simulink model "ofdm_txrx_supermimo"  (Simulink 7.1)
settings: Solver=VariableStepDiscrete  SolverMode=SingleTasking  StartTime=0.0  StopTime=simOnly_simLength  RelTol=1e-3
PostLoadFcn:
  | ofdm_rx_supermimo_init
InitFcn:
  | ofdm_rx_supermimo_init
StartFcn:
  | ofdm_rx_supermimo_init
values from neighbouring files (symbols this model uses):
  Action0_Config = 0* 31*2^18 + 0*2^24 + PHY_AUTORESPONSE_REQ_GOODHDR + PHY_AUTORESPONSE_REQ_GOODPKT + PHY_AUTORESPONSE_REQ_FLAGA    [ofdm_tx_supermimo_init.m]
  Action1_Config = 0* 62*2^18 + PHY_AUTORESPONSE_REQ_GOODHDR + PHY_AUTORESPONSE_REQ_GOODPKT    [ofdm_tx_supermimo_init.m]
  Action2_Config = 0    [ofdm_tx_supermimo_init.m]
  Action3_Config = 0    [ofdm_tx_supermimo_init.m]
  Action4_Config = 0    [ofdm_tx_supermimo_init.m]
  Action5_Config = 0    [ofdm_tx_supermimo_init.m]
  ActionID_AFTransmit = 31    [ofdm_tx_supermimo_init.m]
  ActionID_Disabled = 0    [ofdm_tx_supermimo_init.m]
  ActionID_SetFlagA = 62    [ofdm_tx_supermimo_init.m]
  ActionID_SetFlagB = 61    [ofdm_tx_supermimo_init.m]
  BER_RAM_init_size = 2048    [ofdm_tx_supermimo_init.m]
  CPLength = 16    [ofdm_tx_supermimo_init.m]
  longCorr_coef_i = [-4*real(LTS) 0]    [ofdm_rx_supermimo_init.m]
  longCorr_coef_q = [4*imag(LTS) 0]    [ofdm_rx_supermimo_init.m]
  Match0_Config = 0    [ofdm_tx_supermimo_init.m]
  Match1_Config = 0    [ofdm_tx_supermimo_init.m]
  Match2_Config = 0    [ofdm_tx_supermimo_init.m]
  Match3_Config = 0    [ofdm_tx_supermimo_init.m]
  Match4_Config = 0    [ofdm_tx_supermimo_init.m]
  Match5_Config = 0    [ofdm_tx_supermimo_init.m]
  Match6_Config = 0    [ofdm_tx_supermimo_init.m]
  Match7_Config = 0    [ofdm_tx_supermimo_init.m]
  max_CP_length = 16    [ofdm_tx_supermimo_init.m]
  max_num_baseRateSymbols = 31    [ofdm_tx_supermimo_init.m]
  max_num_subcarriers = 64    [ofdm_tx_supermimo_init.m]
  max_num_trainingSymbols = 15    [ofdm_tx_supermimo_init.m]
  max_numBytes = 16384    [ofdm_tx_supermimo_init.m]
  max_OFDM_symbols = 511    [ofdm_tx_supermimo_init.m]
  modConstellation_qam16 = .75*[1 3 -1 -3]./3    [ofdm_tx_supermimo_init.m]
  modConstellation_qam64 = 0.875*[3 1 5 7 -3 -1 -5 -7]./7    [ofdm_tx_supermimo_init.m]
  modConstellation_qpsk = [1 -1]./sqrt(2)    [ofdm_tx_supermimo_init.m]
  numBaseRateSymbols = ceil(numHeaderBytes / numBytes_BaseRateOFDMSymbol)    [ofdm_tx_supermimo_init.m]
  numSubcarriers = 64    [ofdm_tx_supermimo_init.m]
  numTrainingSymbols = 2    [ofdm_tx_supermimo_init.m]
  pilot_indicies = 7 + ( (64-21) * 2^8) + ( (64-7) * 2^16) + (21 * 2^24)    (= 356068103)    [ofdm_tx_supermimo_init.m]
  pilotValues = hex2dec('A57D') + (2^16 * hex2dec('5A82'))    [ofdm_tx_supermimo_init.m]
  pkt_crossCorr_thresh = 0.7    [ofdm_rx_supermimo_init.m]
  pkt_energy_thresh = 0    [ofdm_rx_supermimo_init.m]
  pktByteNums = numHeaderBytes +  				byteIndex_numPayloadBytes(1)*2^8 +  				byteIndex_numPayloadBytes(2)*2^16 +  				byteIndex_simpleDynModMasks * 2^24    [ofdm_rx_supermimo_init.m]
  PktDet_Delay = 58+32 +      Symbol_Timing_Offset*2^7 +      0*2^16 +      (117* 2^24)    (= 1962935642)    [ofdm_rx_supermimo_init.m]
  pktTiming_count_payloadStart = pktTiming_count_lsStart + pktTiming_ls_num*pktTiming_ls_length    [ofdm_rx_supermimo_init.m]
  preamble = preamble_scale*[shortsyms_10 longsyms_2]    [ofdm_tx_supermimo_init.m]
  preamble_ROM = preamble_I_ROM + preamble_Q_ROM*2^16    [ofdm_tx_supermimo_init.m]
  RAM_init_size = max_numBytes    (= 16384)    [ofdm_tx_supermimo_init.m]
  reg_AF_Tx_Blanking = (320+80) + ( (320+80+79)*2^16)    (= 31392144)    [ofdm_rx_supermimo_init.m]
  reg_AF_Tx_Scaling = 2944    [ofdm_rx_supermimo_init.m]
  reg_Interrupt_PktBuf_Control = reg_InterruptControl +  	1 * 2^16 +  	0 * 2^24    (= 65656)    [ofdm_rx_supermimo_init.m]
  reg_PilotCalcParams = (4 * 2^16) + (minPilotChanMag)    (= 262184.96)    [ofdm_rx_supermimo_init.m]
  regRx_chanEst_minMag = ((0.5)*2^15) + ((0.5 * 2^15)*2^16)    (= 1073758208)    [ofdm_rx_supermimo_init.m]
  regRx_coarseCalcCorrection = round((1.3e-3 - 1.2982e-3)*2^32)    [ofdm_rx_supermimo_init.m]
  regRx_pilotCalcCorrection = round(1.00115 * 2^31)    [ofdm_rx_supermimo_init.m]
  regRx_preCFOoptions = 0 * 2^0 +  	1 * 2^1 +  	0    (= 2)    [ofdm_rx_supermimo_init.m]
  rx_controlBits = 1 * 2  		 + 1 * 4  		 + 0 * 8  		 + 1 * 16 * rx_SISO_Mode  		 + 1 * 32  		 + 1 * 64  		 + 1 * 128  		 + 1 * 256  		 + 1 * 512  		 + 1 * 1024  		 + 1 * 4096  		 + 0 * 16384  		 + 0 * 32768  		 + 1 * 65536  		 + 0 * 131072  		 + 0 * 262144  		 + 1 * 524288  		 + 1 * 1048576 * tx_Alamouti_Mode  		 + 0 * 2097152           + 1 * 33554432  		 + 0 * 4194304  		 + 0 * 8388608  		 + 0 * 16777216  		 + 1 *  ...(+49 chars)    [ofdm_rx_supermimo_init.m]
  Rx_PktDet_LongCorr_Params = LongCorrCounterLoad + 0*2^8 + 0*2^16 + 255*2^24    (= 4278190325)    [ofdm_rx_supermimo_init.m]
  Rx_PktDet_LongCorrThresholds = 8000    [ofdm_rx_supermimo_init.m]
  rx_postEq_scaling = round(rxScaling*2^11) * (1 + 2^16)    [ofdm_rx_supermimo_init.m]
  RxDataScrambling_Seq = [40 198 78 63 82 173 102 245 48 111 172 115 147 230 216 93 72 65 62 2 205 242 122 90 128 83 105 97 73 10 5 252]    [ofdm_tx_supermimo_init.m]
  RxReg_FixedPktLen = 0    [ofdm_rx_supermimo_init.m]
  shortSymbol_time = shortSymbol_time(1:16).'    [ofdm_tx_supermimo_init.m]
  simOnly_numSamples = length(preamble)+( (numSubcarriers+CPLength)*(numTrainingSymbols + numBaseRateSymbols + numFullRateSymbols/2) )    [ofdm_tx_supermimo_init.m]
  train = [train train]    [ofdm_tx_supermimo_init.m]
  tx_controlBits = (antB_preambleShift * 2^4) + (2^1 * tx_Alamouti_Mode) +  	(2^0 * tx_SISO_Mode) + 4*0 + 8*1 + 0*256 + 512*0 + 1024*0 +  	0*hex2dec('1000') + 0*hex2dec('2000') + 0*hex2dec('4000') +  	1*hex2dec('8000') + 1*hex2dec('10000') + hex2dec('20000') +  	16*hex2dec('100000')    [ofdm_tx_supermimo_init.m]
  tx_delays = (50*2^0) + (0*2^8) + (30*2^12)    (= 122930)    [ofdm_tx_supermimo_init.m]
  tx_scaling = (3072) + (12288 * 2^16)    (= 805309440)    [ofdm_tx_supermimo_init.m]
  TxDataScrambling_Seq = [40 198 78 63 82 173 102 245 48 111 172 115 147 230 216 93 72 65 62 2 205 242 122 90 128 83 105 97 73 10 5 252]    [ofdm_tx_supermimo_init.m]
  txfilt_firpm = 0.95*txfilt_firpm./(max(txfilt_firpm))    [ofdm_tx_supermimo_init.m]
  txfilt_intfilt = [0 intfilt(4,4,.8)]    [ofdm_tx_supermimo_init.m]
  txReg_symbolCounts = (2^8 *numBaseRateSymbols ) + numTrainingSymbols    [ofdm_tx_supermimo_init.m]
  TxRx_FFTScaling = (3 * 2^0) +  	(2 * 2^2) +  	(1 * 2^4) +  	(1 * 2^6) +  	(1 * 2^8) +  	(0 * 2^10)    (= 347)    [ofdm_tx_supermimo_init.m]
undefined symbols (not defined in the file or in its folder): AntA, AntB, BaseRate, Down, gcbh, numBuffs, numBytes, Up, xlBlockLoadCallback, xlGetSimulinkPeriod, xlipgetpartsetting
libraries: xbsIndex_r4 x6035, simulink x4, dspsigops x4, commchan3 x2, dspsrcs4 x2, commrflib2 x2
(part 13 of 61)

== system ofdm_txrx_supermimo/OFDM Rx MIMO/FFT & Chan Est/Addr_Generator ==
  note: The FFT core begins requesting input samples immediately
      | after its Start input is asserted. If Start is asserted again before
      | the first transform is complete, a second transform begins
      | immediately after the first. This is how we sequence the pairs
      | of transforms for the incoming OFDM symbols multiplexed between
      | antennas. The FFT is started as soon as the incoming sample stream
      | passes sample number (numSubcarriers/2)+symbolTimingOffset.
      | This is the lowest latency scheme, loading the FFT core with the
      | input OFDM symbol exactly 1 clock cycle after it's available.
  note: The memory read address is the FFT input index plus an offset in [0,max_cyclic_prefix]. This is how we strip
      | the cyclic prefix from the incoming sample stream- the FFT only gets numSubcarrier samples.
  Payload : Inport  OutDataType=sfix(16)  OutScaling=2^0
  FFT Xn_index : Inport  Port=2  OutDataType=sfix(16)  OutScaling=2^0
  AddSub : xbsIndex_r4/AddSub  mode=Addition  use_carryin=off  use_carryout=off  en=off  latency=0  precision=User Defined  arith_type=Unsigned  n_bits=ceil(log2(max_num_subcarriers))+1  bin_pt=0  quantization=Truncate  overflow=Wrap  dbl_ovrd=off  use_behavioral_HDL=on  pipelined=off  use_rpm=on  hw_selection=Fabric  block_type=addsub
      settings: xl_area=[4 0 0 6 0 0 0]  block_version=VER_STRING_GOES_HERE
  Assert : xbsIndex_r4/Assert  Orientation=left  assert_type=on  type_source=Explicitly  arith_type=Boolean  n_bits=16  bin_pt=14  assert_rate=off  rate_source=Explicitly  period=1  output_port=on  block_type=assert
      settings: infoedit=Asserts a user-defined sample rate and/or type on a signal.<P><P>Hardware notes: In hardware this block costs nothing.  block_version=VER_STRING_GOES_HERE
  Convert : xbsIndex_r4/Convert  arith_type=Unsigned  n_bits=ceil(log2(max_num_subcarriers))+1  bin_pt=0  quantization=Truncate  overflow=Wrap  latency=0  dbl_ovrd=off  pipeline=off  block_type=convert
      settings: block_version=VER_STRING_GOES_HERE
  Delay : xbsIndex_r4/Delay  en=off  latency=64  dbl_ovrd=off  reg_retiming=off  block_type=delay
      settings: infoedit=Hardware notes: A delay line is a chain, each link of which is an SRL16 followed by a flip-flop. If register retiming is enabled, the delay line is a chain of flip-flops.  block_version=VER_STRING_GOES_HERE
  Down Sample : xbsIndex_r4/Down Sample  Orientation=left  sample_ratio=2  sample_phase="Last Value of Frame  (most efficient)"  en=off  latency=1  dbl_ovrd=off  block_type=dsamp
      settings: block_version=VER_STRING_GOES_HERE
  Initial Read Addr Timing Calc : Subsystem
  Inverter : xbsIndex_r4/Inverter  Orientation=left  en=off  latency=0  dbl_ovrd=off  block_type=inv
      settings: block_version=VER_STRING_GOES_HERE
  Inverter1 : xbsIndex_r4/Inverter  en=off  latency=0  dbl_ovrd=off  block_type=inv
      settings: block_version=VER_STRING_GOES_HERE
  Logical1 : xbsIndex_r4/Logical  logical_function=OR  inputs=3  en=off  latency=0  precision=Full  arith_type=Unsigned  n_bits=16  bin_pt=0  align_bp=on  dbl_ovrd=off  block_type=logical
      settings: block_version=VER_STRING_GOES_HERE
  Logical2 : xbsIndex_r4/Logical  logical_function=OR  inputs=2  en=off  latency=0  precision=Full  arith_type=Unsigned  n_bits=16  bin_pt=0  align_bp=on  dbl_ovrd=off  block_type=logical
      settings: block_version=VER_STRING_GOES_HERE
  Logical3 : xbsIndex_r4/Logical  logical_function=OR  inputs=2  en=off  latency=0  precision=Full  arith_type=Unsigned  n_bits=16  bin_pt=0  align_bp=on  dbl_ovrd=off  block_type=logical
      settings: block_version=VER_STRING_GOES_HERE
  Logical4 : xbsIndex_r4/Logical  logical_function=AND  inputs=3  en=off  latency=0  precision=Full  arith_type=Unsigned  n_bits=16  bin_pt=0  align_bp=on  dbl_ovrd=off  block_type=logical
      settings: block_version=VER_STRING_GOES_HERE
  Logical5 : xbsIndex_r4/Logical  logical_function=OR  inputs=2  en=off  latency=0  precision=Full  arith_type=Unsigned  n_bits=16  bin_pt=0  align_bp=on  dbl_ovrd=off  block_type=logical
      settings: block_version=VER_STRING_GOES_HERE
  MSB : xbsIndex_r4/Slice  nbits=1  boolean_output=on  mode=Upper Bit Location + Width  bit1=0  base1=MSB of Input  bit0=0  base0=LSB of Input  dbl_ovrd=off  block_type=slice
      settings: infoedit="Extracts a given range of bits from each input sample and presents it at the output.  The output type is ordinarily unsigned with binary point at zero, but can be Boolean when the slice is one bit wide.<P><P>Hardware notes: In hardware this block costs nothing."  block_version=VER_STRING_GOES_HERE
  Oscillator : Subsystem
  Posedge : Subsystem
  Register : xbsIndex_r4/Register  init=0  rst=on  en=on  dbl_ovrd=off  block_type=register
      settings: block_version=VER_STRING_GOES_HERE
  Relational : xbsIndex_r4/Relational  Orientation=left  mode=a=b  en=off  latency=0  dbl_ovrd=off  block_type=relational
      settings: block_version=VER_STRING_GOES_HERE
  Relational1 : xbsIndex_r4/Relational  mode=a=b  en=off  latency=0  dbl_ovrd=off  block_type=relational
      settings: block_version=VER_STRING_GOES_HERE
  Samp Counter : xbsIndex_r4/Counter  cnt_type=Free Running  cnt_to=1  operation=Up  start_count=0  cnt_by_val=1  arith_type=Unsigned  n_bits=ceil(log2(max_num_subcarriers + max_CP_length))  bin_pt=0  load_pin=off  rst=on  en=on  explicit_period=off  period=1  dbl_ovrd=off  use_behavioral_HDL=off  use_rpm=on  implementation=Fabric  block_type=counter
      settings: xl_area=[5 7 0 9 0 0 0]  block_version=VER_STRING_GOES_HERE
  Up Sample1 : xbsIndex_r4/Up Sample  sample_ratio=4  copy_samples=on  dbl_ovrd=off  block_type=usamp
      settings: infoedit="Up samples input data.  Inserted values  can be zeros or copies of the most recent input sample.<P><P>Hardware notes: No hardware is needed if inserted values are copies of the input sample; otherwise, a mux and single bit flip-flop are used."  block_version=VER_STRING_GOES_HERE
  Up Sample2 : xbsIndex_r4/Up Sample  sample_ratio=4  copy_samples=on  dbl_ovrd=off  block_type=usamp
      settings: infoedit="Up samples input data.  Inserted values  can be zeros or copies of the most recent input sample.<P><P>Hardware notes: No hardware is needed if inserted values are copies of the input sample; otherwise, a mux and single bit flip-flop are used."  block_version=VER_STRING_GOES_HERE
  Up Sample6 : xbsIndex_r4/Up Sample  sample_ratio=2  copy_samples=on  dbl_ovrd=off  block_type=usamp
      settings: infoedit="Up samples input data.  Inserted values  can be zeros or copies of the most recent input sample.<P><P>Hardware notes: No hardware is needed if inserted values are copies of the input sample; otherwise, a mux and single bit flip-flop are used."  block_version=VER_STRING_GOES_HERE
  negedge : Subsystem
  negedge1 : Subsystem
  Write Addr : Outport  OutDataType=sfix(16)  OutScaling=2^0
  Read Addr : Outport  Port=2  OutDataType=sfix(16)  OutScaling=2^0
  Ant Sel : Outport  Port=3  OutDataType=sfix(16)  OutScaling=2^0
  Wr Slot : Outport  Port=4  OutDataType=sfix(16)  OutScaling=2^0
  FFT Start : Outport  Port=5  OutDataType=sfix(16)  OutScaling=2^0
  wires:
  negedge1/1(Q) -> Logical1/1
  Up Sample2/1 -> Logical4/2
  Logical4/1 -> FFT Start/1
  [localReset] -> Register/2
  Assert/1 -> Inverter/1
  Inverter/1 -> Register/1
  Register/1 -> Assert/1, Ant Sel/1
  negedge/1(Q) -> Register/3
  MSB/1 -> negedge/1(D)
  Logical2/1 -> Logical4/3
  Up Sample1/1 -> Relational1/1
  FFT Xn_index/1 -> Convert/1, MSB/1
  Down Sample/1 -> Relational/1
  Relational1/1 -> Logical2/1, Delay/1
  AddSub/1 -> Read Addr/1
  [rx_global_reset_DV2] -> Logical1/3
  [localReset] -> Logical3/2, Oscillator/2(rst)
  Logical3/1 -> Samp Counter/1
  Payload/1 -> negedge1/1(D), Samp Counter/2, Up Sample2/1, Posedge/1(D)
  Logical1/1 -> [localReset]
  Posedge/1(Q) -> Logical1/2
  Relational/1 -> Logical3/1, Oscillator/1(en)
  Samp Counter/1 -> Up Sample1/1, Write Addr/1, Relational/2
  [regRX_sampsPerSymMinusOne] -> Down Sample/1
  [regRx_symbolTimingOffset] -> Up Sample6/1
  Convert/1 -> AddSub/1
  Up Sample6/1 -> AddSub/2
  Initial Read Addr Timing Calc/1(D) -> Relational1/2
  Delay/1 -> Logical2/2
  Oscillator/1(Q) -> Wr Slot/1, Logical5/2
  Logical5/1 -> Logical4/1
  [regRx_AlamoutiMode] -> Inverter1/1
  Inverter1/1 -> Logical5/1

== system ofdm_txrx_supermimo/OFDM Rx MIMO/FFT & Chan Est/Channel Estimation & Pilot Processing ==
  note: Naming conventions:
      | Antennas are labaled A and B
      | H_A-A is channel between TxA and RxA
      | H_A-B is channel between TxA and RxB
      | H_B-A is channel between TxB and RxA
      | H_B-B is channel between TxB and RxB
  I : Inport  OutDataType=sfix(16)  OutScaling=2^0
  Q : Inport  Port=2  OutDataType=sfix(16)  OutScaling=2^0
  Xk Index : Inport  Port=3  OutDataType=sfix(16)  OutScaling=2^0
  Vin : Inport  Port=4  OutDataType=sfix(16)  OutScaling=2^0
  Sym Index : Inport  Port=5  OutDataType=sfix(16)  OutScaling=2^0
  Est Index : Inport  Port=6  OutDataType=sfix(16)  OutScaling=2^0
  Actual Trainig Window : Subsystem
  Bus Creator : Subsystem
  Chan Est Buffer & Mag Calc : Subsystem
  Delay1 : xbsIndex_r4/Delay  en=off  latency=1  dbl_ovrd=off  reg_retiming=on  block_type=delay
      settings: infoedit=Hardware notes: A delay line is a chain, each link of which is an SRL16 followed by a flip-flop. If register retiming is enabled, the delay line is a chain of flip-flops.  xl_area=[0, 0, 0, 0, 0, 0, 0]  block_version=VER_STRING_GOES_HERE
  Delay{2,4..11} (9 alike) : xbsIndex_r4/Delay  en=off  latency=1  dbl_ovrd=off  reg_retiming=off  block_type=delay
      settings: infoedit=Hardware notes: A delay line is a chain, each link of which is an SRL16 followed by a flip-flop. If register retiming is enabled, the delay line is a chain of flip-flops.  xl_area=[0, 0, 0, 0, 0, 0, 0]  block_version=VER_STRING_GOES_HERE
  Delay3 : xbsIndex_r4/Delay  en=off  latency=1  dbl_ovrd=off  reg_retiming=on  block_type=delay
      settings: infoedit=Hardware notes: A delay line is a chain, each link of which is an SRL16 followed by a flip-flop. If register retiming is enabled, the delay line is a chain of flip-flops.  xl_area=[0, 0, 0, 0, 0, 0, 0]  block_version=VER_STRING_GOES_HERE
  Down Sample : xbsIndex_r4/Down Sample  sample_ratio=2  sample_phase="Last Value of Frame  (most efficient)"  en=off  latency=1  dbl_ovrd=off  block_type=dsamp
      settings: block_version=10.1.2
  Downsamplng : Subsystem
  H_A-A Estimate Buffer : Subsystem
  H_A-B Estimate Buffer : Subsystem
  H_B-A Estimate Buffer : Subsystem
  H_B-B Estimate Buffer : Subsystem
  Inverter : xbsIndex_r4/Inverter  en=off  latency=1  dbl_ovrd=off  block_type=inv
      settings: block_version=VER_STRING_GOES_HERE
  Logical1 : xbsIndex_r4/Logical  logical_function=OR  inputs=2  en=off  latency=0  precision=Full  arith_type=Unsigned  n_bits=8  bin_pt=2  align_bp=on  dbl_ovrd=off  block_type=logical
      settings: xl_area=[1 0 0 1 0 0 0]  block_version=VER_STRING_GOES_HERE
  Logical2 : xbsIndex_r4/Logical  logical_function=OR  inputs=2  en=off  latency=0  precision=Full  arith_type=Unsigned  n_bits=8  bin_pt=2  align_bp=on  dbl_ovrd=off  block_type=logical
      settings: xl_area=[1 0 0 1 0 0 0]  block_version=VER_STRING_GOES_HERE
  Mult by training : Subsystem
  Mux3 : xbsIndex_r4/Mux  inputs=2  en=off  latency=0  precision=Full  arith_type="Signed  (2's comp)"  n_bits=8  bin_pt=2  quantization=Truncate  overflow=Wrap  dbl_ovrd=off  block_type=mux
      settings: xl_area=[0, 0, 0, 0, 0, 0, 0]  block_version=VER_STRING_GOES_HERE
  Pilot Processing : Subsystem
  Training Symbol : xbsIndex_r4/ROM  depth=length(train)/2  initVector=sign(train)  distributed_mem=Block RAM  rst=off  init_reg=0  en=off  latency=1  arith_type="Signed  (2's comp)"  n_bits=2  bin_pt=1  dbl_ovrd=off  optimize=Area  use_rpm=off  block_type=sprom
      settings: xl_area=[0, 0, 0, 0, 0, 0, 0]  block_version=VER_STRING_GOES_HERE
  Training? : Subsystem
  WE Gen : Subsystem
  done{1..9,23} (10 alike) : xbsIndex_r4/Gateway Out  hdl_port=off  timing_constraint=None  locs_specified=off  LOCs={}  block_type=gatewayout
      settings: infoedit="Gateway out block.  Converts Xilinx fixed point inputs into ouputs of type Simulink integer, double, or fixed point.<P><P>Hardware notes:  In hardware these blocks become top level output ports or are discarded, depending on how they are configured."  xl_area=[0 0 0 0 1 0 0]  block_version=VER_STRING_GOES_HERE
  H-I : Outport  OutDataType=sfix(16)  OutScaling=2^0
  H-Q : Outport  Port=2  OutDataType=sfix(16)  OutScaling=2^0
  Phase I : Outport  Port=3  OutDataType=sfix(16)  OutScaling=2^0
  Phase Q : Outport  Port=4  OutDataType=sfix(16)  OutScaling=2^0
  Est Vout : Outport  Port=5  OutDataType=sfix(16)  OutScaling=2^0
  wires:
  Delay5/1 -> H_A-B Estimate Buffer/4(Est_Reset), H_B-B Estimate Buffer/4(Est_Reset), Logical1/2, Logical2/2
  Delay2/1 -> Mux3/3, H_A-A Estimate Buffer/5(FFT_Addr), Pilot Processing/3(Xk_Index), H_B-A Estimate Buffer/5(FFT_Addr)
  Est Index/1 -> Mux3/2, Delay7/1, Chan Est Buffer & Mag Calc/10(SubCar Index)
  Delay10/1 -> Delay11/1
  done23/1 -> ?
  done9/1 -> ?
  done2/1 -> ?
  done8/1 -> ?
  done7/1 -> ?
  done6/1 -> ?
  done3/1 -> ?
  done5/1 -> ?
  done4/1 -> ?
  done1/1 -> ?
  H_B-B Estimate Buffer/2(EstQ) -> Downsamplng/8(BB-Q)
  H_B-B Estimate Buffer/1(EstI) -> Downsamplng/7(BB-I)
  H_B-A Estimate Buffer/4(EstQ) -> Downsamplng/6(BA-Q)
  H_B-A Estimate Buffer/3(EstI) -> Downsamplng/5(BA-I)
  H_A-B Estimate Buffer/2(EstQ) -> Downsamplng/4(AB-Q)
  H_A-B Estimate Buffer/1(EstI) -> Downsamplng/3(AB-I)
  H_A-A Estimate Buffer/4(EstQ) -> Downsamplng/2(AA-Q)
  H_A-A Estimate Buffer/3(EstI) -> Downsamplng/1(AA-I)
  Delay11/1 -> Down Sample/1
  Inverter/1 -> Delay10/1
  Delay7/1 -> done2/1
  Delay9/1 -> WE Gen/1(Vin)
  Vin/1 -> Delay9/1, Actual Trainig Window/1(H Vin)
  Delay8/1 -> Mult by training/2(AI)
  Delay6/1 -> Mult by training/1(AR)
  Delay3/1 -> Mux3/1, WE Gen/3(Train), Actual Trainig Window/2(Train Sym)
  Delay1/1 -> WE Gen/2(Sym Index), Pilot Processing/4(Sym_Index)
  Mult by training/2(PI) -> H_A-B Estimate Buffer/2(Q), H_A-A Estimate Buffer/2(Q), H_B-B Estimate Buffer/2(Q), H_B-A Estimate Buffer/2(Q)
  Training?/2(Training) -> Delay3/1, Inverter/1
  WE Gen/2(Seq1) -> H_A-B Estimate Buffer/3(Est_WE)
  WE Gen/4(Seq3) -> H_B-B Estimate Buffer/3(Est_WE)
  WE Gen/3(Seq2) -> H_B-A Estimate Buffer/3(Est_WE)
  WE Gen/1(Seq0) -> H_A-A Estimate Buffer/3(Est_WE)
  Mux3/1 -> H_B-B Estimate Buffer/5(Xk_Index), H_A-B Estimate Buffer/5(Xk_Index), H_A-A Estimate Buffer/6(EQ_Addr), H_B-A Estimate Buffer/6(EQ_Addr)
  Mult by training/1(PR) -> H_A-B Estimate Buffer/1(I), H_A-A Estimate Buffer/1(I), H_B-A Estimate Buffer/1(I), H_B-B Estimate Buffer/1(I)
  Xk Index/1 -> Training Symbol/1, Delay4/1
  Sym Index/1 -> Training?/1(SynIndex), Delay1/1
  Q/1 -> Delay8/1, Pilot Processing/2(Q)
  I/1 -> Delay6/1, Pilot Processing/1(I)
  Delay4/1 -> Delay2/1
  Training Symbol/1 -> Mult by training/3(BR)
  Down Sample/1 -> Est Vout/1, Chan Est Buffer & Mag Calc/9(Est Vin), done23/1
  Bus Creator/1(I) -> H-I/1
  Bus Creator/2(Q) -> H-Q/1
  Downsamplng/1(" AA-I") -> Bus Creator/1(AA-I), Chan Est Buffer & Mag Calc/1(AA-I), done9/1
  Downsamplng/2(" AA-Q") -> Bus Creator/2(AA-Q), Chan Est Buffer & Mag Calc/2(AA-Q), done4/1
  Downsamplng/3(" AB-I") -> Bus Creator/3(AB-I), Chan Est Buffer & Mag Calc/3(AB-I), done5/1
  Downsamplng/4(" AB-Q") -> Bus Creator/4(AB-Q), Chan Est Buffer & Mag Calc/4(AB-Q), done3/1
  Downsamplng/5(" BA-I") -> Bus Creator/5(BA-I), Chan Est Buffer & Mag Calc/5(BA-I), done6/1
  Downsamplng/6(" BA-Q") -> Bus Creator/6(BA-Q), Chan Est Buffer & Mag Calc/6(BA-Q), done1/1
  Downsamplng/7(" BB-I") -> Bus Creator/7(BB-I), Chan Est Buffer & Mag Calc/7(BB-I), done7/1
  Downsamplng/8(" BB-Q") -> Bus Creator/8(BB-Q), Chan Est Buffer & Mag Calc/8(BB-Q), done8/1
  Training?/1(FirstSym) -> Delay5/1
  Pilot Processing/1(Phase I) -> Phase I/1
  H_A-A Estimate Buffer/1(EstI_fast) -> Pilot Processing/6(HAA_I)
  H_A-A Estimate Buffer/2(EstQ_fast) -> Pilot Processing/7(HAA_Q)
  H_B-A Estimate Buffer/2(EstQ_fast) -> Pilot Processing/9(HBA_Q)
  H_B-A Estimate Buffer/1(EstI_fast) -> Pilot Processing/8(HBA_I)
  Actual Trainig Window/1(Train) -> Pilot Processing/5(Train)
  Pilot Processing/2(Phase Q) -> Phase Q/1
  Logical2/1 -> H_A-A Estimate Buffer/4(Est_Reset)
  [ChanEstMagCheck_MaskAA] -> Logical2/1
  Logical1/1 -> H_B-A Estimate Buffer/4(Est_Reset)
  [ChanEstMagCheck_MaskBA] -> Logical1/1

== system ofdm_txrx_supermimo/OFDM Rx MIMO/FFT & Chan Est/FFT ==
  xn_I : Inport  OutDataType=sfix(16)  OutScaling=2^0
  xn_Q : Inport  Port=2  OutDataType=sfix(16)  OutScaling=2^0
  Start : Inport  Port=3  OutDataType=sfix(16)  OutScaling=2^0
  Constant3 : xbsIndex_r4/Constant  arith_type=Boolean  const=1  n_bits=1  bin_pt=0  explicit_period=off  period=1  equ=P=C  opselect=C  inp2=PCIN>>17  opr=+  inp1=P  carry=CIN  dbl_ovrd=off  block_type=constant
      settings: block_version=VER_STRING_GOES_HERE
  Constant4 : xbsIndex_r4/Constant  arith_type=Boolean  const=1  n_bits=1  bin_pt=0  explicit_period=off  period=1  equ=P=C  opselect=C  inp2=PCIN>>17  opr=+  inp1=P  carry=CIN  dbl_ovrd=off  block_type=constant
      settings: block_version=VER_STRING_GOES_HERE
  Constant6 : xbsIndex_r4/Constant  arith_type=Boolean  const=1  n_bits=1  bin_pt=0  explicit_period=off  period=1  equ=P=C  opselect=C  inp2=PCIN>>17  opr=+  inp1=P  carry=CIN  dbl_ovrd=off  block_type=constant
      settings: block_version=VER_STRING_GOES_HERE
  Convert : xbsIndex_r4/Convert  arith_type="Signed  (2's comp)"  n_bits=16  bin_pt=15  quantization=Truncate  overflow=Flag as error  latency=0  dbl_ovrd=off  pipeline=off  block_type=convert
      settings: block_version=VER_STRING_GOES_HERE
  Convert1 : xbsIndex_r4/Convert  arith_type="Signed  (2's comp)"  n_bits=16  bin_pt=15  quantization=Truncate  overflow=Flag as error  latency=0  dbl_ovrd=off  pipeline=off  block_type=convert
      settings: block_version=VER_STRING_GOES_HERE
  "Fast Fourier Transform 6.0 " : xbsIndex_r4/Fast Fourier Transform 6.0   transform_length=64  implementation_options=pipelined_streaming_io  run_time_configurable_transform_length=off  phase_factor_width=24  scaling_options=scaled  rounding_modes=convergent_rounding  output_ordering=natural_order  cyclic_prefix_insertion=off  en=off  rst=on  ovflo=on  memory_options_data=block_ram  memory_options_phase_factors=block_ram  number_of_stages_using_block_ram_for_data_and_phase_factors=1  memory_options_reorder=block_ram  memory_options_hybrid=off  optimize_for_speed_using_xtreme_dsp_slices=off  fast_complex_mult=off  fast_butterfly=off  target_clock_frequency=250  simulation_type=external_xfix  simulation_model=fftv6_CModel:fftv6_cmodel  ip_name=Fast Fourier Transform  ip_version=6.0  dsptool_ready=true  input_ordering=natural_order  ce=true  sclr=0  wrapper_available=true  port_translation_map={ 'ce' => 'en', 'sclr' => 'rst' }  ipcore_fpga_part=xlipgetpartsetting(gcb, {'virtex4', 'xc4vsx35', '-10', 'ff668'})  target_data_throughput=50  channels=1  input_width=16  block_type=xfft_v6_0
      settings: block_version=10.1.3
  "FreqSelective \"Fading\"" : Subsystem
  Up Sample1 : xbsIndex_r4/Up Sample  sample_ratio=2  copy_samples=on  dbl_ovrd=off  block_type=usamp
      settings: infoedit="Up samples input data.  Inserted values  can be zeros or copies of the most recent input sample.<P><P>Hardware notes: No hardware is needed if inserted values are copies of the input sample; otherwise, a mux and single bit flip-flop are used."  block_version=VER_STRING_GOES_HERE
  Up Sample2 : xbsIndex_r4/Up Sample  sample_ratio=2  copy_samples=on  dbl_ovrd=off  block_type=usamp
      settings: infoedit="Up samples input data.  Inserted values  can be zeros or copies of the most recent input sample.<P><P>Hardware notes: No hardware is needed if inserted values are copies of the input sample; otherwise, a mux and single bit flip-flop are used."  block_version=VER_STRING_GOES_HERE
  xk_I : Outport  OutDataType=sfix(16)  OutScaling=2^0
  xk_Q : Outport  Port=2  OutDataType=sfix(16)  OutScaling=2^0
  xk_Index : Outport  Port=3  OutDataType=sfix(16)  OutScaling=2^0
  Vout : Outport  Port=4  OutDataType=sfix(16)  OutScaling=2^0
  Done : Outport  Port=5  OutDataType=sfix(16)  OutScaling=2^0
  xn_Index : Outport  Port=6  OutDataType=sfix(16)  OutScaling=2^0
  tag scope: FFT_Overflow=global
  wires:
  [Rx_FFTScaling] -> Up Sample2/1
  Constant6/1 -> "Fast Fourier Transform 6.0 "/7
  Convert1/1 -> "Fast Fourier Transform 6.0 "/2
  Convert/1 -> "Fast Fourier Transform 6.0 "/1
  "Fast Fourier Transform 6.0 "/7 -> Vout/1
  "Fast Fourier Transform 6.0 "/4 -> xk_Index/1, "FreqSelective \"Fading\""/3(Ind)
  Start/1 -> "Fast Fourier Transform 6.0 "/3
  "Fast Fourier Transform 6.0 "/3 -> xn_Index/1
  xn_Q/1 -> Convert1/1
  "Fast Fourier Transform 6.0 "/2 -> "FreqSelective \"Fading\""/2(Q)
  "Fast Fourier Transform 6.0 "/1 -> "FreqSelective \"Fading\""/1(I)
  xn_I/1 -> Convert/1
  [rx_global_reset] -> Up Sample1/1
  Constant4/1 -> "Fast Fourier Transform 6.0 "/5
  Constant3/1 -> "Fast Fourier Transform 6.0 "/4
  Up Sample1/1 -> "Fast Fourier Transform 6.0 "/8
  Up Sample2/1 -> "Fast Fourier Transform 6.0 "/6
  "Fast Fourier Transform 6.0 "/8 -> Done/1
  "Fast Fourier Transform 6.0 "/9 -> ?
  "Fast Fourier Transform 6.0 "/10 -> [FFT_Overflow]
  "FreqSelective \"Fading\""/1(" I") -> xk_I/1
  "FreqSelective \"Fading\""/2(" Q") -> xk_Q/1

== system ofdm_txrx_supermimo/OFDM Rx MIMO/FFT & Chan Est/Input Buffers ==
  AntA I : Inport  OutDataType=sfix(16)  OutScaling=2^0
  AntA Q : Inport  Port=2  OutDataType=sfix(16)  OutScaling=2^0
  AntB I : Inport  Port=3  OutDataType=sfix(16)  OutScaling=2^0
  AntB Q : Inport  Port=4  OutDataType=sfix(16)  OutScaling=2^0
  We : Inport  Port=5  OutDataType=sfix(16)  OutScaling=2^0
  Wr Addr : Inport  Port=6  OutDataType=sfix(16)  OutScaling=2^0
  Rd Addr : Inport  Port=7  OutDataType=sfix(16)  OutScaling=2^0
  Ant Sel : Inport  Port=8  OutDataType=sfix(16)  OutScaling=2^0
  Wr Slot : Inport  Port=9  OutDataType=sfix(16)  OutScaling=2^0
  Antenna A RAM : Subsystem
  Antenna B RAM : Subsystem
  Down Sample : xbsIndex_r4/Down Sample  sample_ratio=2  sample_phase="Last Value of Frame  (most efficient)"  en=off  latency=1  dbl_ovrd=off  block_type=dsamp
      settings: block_version=VER_STRING_GOES_HERE
  Mux : xbsIndex_r4/Mux  inputs=2  en=off  latency=1  precision=Full  arith_type=Unsigned  n_bits=16  bin_pt=14  quantization=Truncate  overflow=Wrap  dbl_ovrd=off  block_type=mux
      settings: block_version=VER_STRING_GOES_HERE
  Mux1 : xbsIndex_r4/Mux  inputs=2  en=off  latency=1  precision=Full  arith_type=Unsigned  n_bits=16  bin_pt=14  quantization=Truncate  overflow=Wrap  dbl_ovrd=off  block_type=mux
      settings: block_version=VER_STRING_GOES_HERE
  Mux2 : xbsIndex_r4/Mux  inputs=2  en=off  latency=0  precision=Full  arith_type=Unsigned  n_bits=16  bin_pt=14  quantization=Truncate  overflow=Wrap  dbl_ovrd=off  block_type=mux
      settings: block_version=VER_STRING_GOES_HERE
  Mux3 : xbsIndex_r4/Mux  inputs=2  en=off  latency=0  precision=Full  arith_type=Unsigned  n_bits=16  bin_pt=14  quantization=Truncate  overflow=Wrap  dbl_ovrd=off  block_type=mux
      settings: block_version=VER_STRING_GOES_HERE
  Register : xbsIndex_r4/Register  init=0  rst=off  en=off  dbl_ovrd=off  block_type=register
      settings: block_version=VER_STRING_GOES_HERE
  Register1 : xbsIndex_r4/Register  init=0  rst=off  en=off  dbl_ovrd=off  block_type=register
      settings: block_version=VER_STRING_GOES_HERE
  We Control : Subsystem
  " I" : Outport  OutDataType=sfix(16)  OutScaling=2^0
  " Q" : Outport  Port=2  OutDataType=sfix(16)  OutScaling=2^0
  wires:
  Register1/1 -> " Q"/1
  Register/1 -> " I"/1
  Mux1/1 -> Register1/1
  Mux/1 -> Register/1
  Ant Sel/1 -> Mux/1, Mux1/1
  Antenna B RAM/1(" I") -> Mux/3
  Antenna B RAM/2(" Q") -> Mux1/3
  Antenna A RAM/2(" Q") -> Mux1/2
  Antenna A RAM/1(" I") -> Mux/2
  Rd Addr/1 -> Antenna B RAM/5(Rd Addr), Antenna A RAM/5(Rd Addr)
  Wr Addr/1 -> Antenna B RAM/4(Wr Addr), Antenna A RAM/4(Wr Addr)
  Mux2/1 -> Antenna B RAM/1(I)
  AntB I/1 -> Mux2/2
  AntA I/1 -> Antenna A RAM/1(I), Mux2/3
  AntA Q/1 -> Antenna A RAM/2(Q), Mux3/3
  We Control/1(A_We) -> Antenna A RAM/3(We)
  We Control/2(B_We) -> Antenna B RAM/3(We)
  AntB Q/1 -> Mux3/2
  [regRx_AlamoutiMode] -> Down Sample/1
  Mux3/1 -> Antenna B RAM/2(Q)
  We/1 -> We Control/1(We)
  Wr Slot/1 -> We Control/2(WrSlot)
  Down Sample/1 -> Mux3/1, Mux2/1

== system ofdm_txrx_supermimo/OFDM Rx MIMO/FFT & Chan Est/Output Buffers ==
  I : Inport  OutDataType=sfix(16)  OutScaling=2^0
  Q : Inport  Port=2  OutDataType=sfix(16)  OutScaling=2^0
  Wr Addr : Inport  Port=3  OutDataType=sfix(16)  OutScaling=2^0
  FFT Vout : Inport  Port=4  OutDataType=sfix(16)  OutScaling=2^0
  SymIndex : Inport  Port=5  OutDataType=sfix(16)  OutScaling=2^0
  Rd Addr : Inport  Port=6  OutDataType=sfix(16)  OutScaling=2^0
  AntA Buffer : Subsystem
  AntB Buffer : Subsystem
  Bus Creator1 : BusCreator  Inputs=2
  Bus Creator2 : BusCreator  Inputs=2
  Inverter : xbsIndex_r4/Inverter  en=off  latency=0  dbl_ovrd=off  block_type=inv
      settings: block_version=VER_STRING_GOES_HERE
  LSB : xbsIndex_r4/Slice  nbits=1  boolean_output=on  mode=Upper Bit Location + Width  bit1=0  base1=LSB of Input  bit0=0  base0=LSB of Input  dbl_ovrd=off  block_type=slice
      settings: infoedit="Extracts a given range of bits from each input sample and presents it at the output.  The output type is ordinarily unsigned with binary point at zero, but can be Boolean when the slice is one bit wide.<P><P>Hardware notes: In hardware this block costs nothing."  block_version=VER_STRING_GOES_HERE
  Logical1 : xbsIndex_r4/Logical  logical_function=AND  inputs=2  en=off  latency=0  precision=Full  arith_type=Unsigned  n_bits=8  bin_pt=2  align_bp=on  dbl_ovrd=off  block_type=logical
      settings: xl_area=[0, 0, 0, 0, 0, 0, 0]  block_version=VER_STRING_GOES_HERE
  Logical2 : xbsIndex_r4/Logical  logical_function=AND  inputs=2  en=off  latency=0  precision=Full  arith_type=Unsigned  n_bits=8  bin_pt=2  align_bp=on  dbl_ovrd=off  block_type=logical
      settings: xl_area=[0, 0, 0, 0, 0, 0, 0]  block_version=VER_STRING_GOES_HERE
  " I" : Outport  OutDataType=sfix(16)  OutScaling=2^0
  " Q" : Outport  Port=2  OutDataType=sfix(16)  OutScaling=2^0
  wires:
  Bus Creator2/1 -> " Q"/1
  Bus Creator1/1 -> " I"/1
  Inverter/1 -> Logical2/1
  Q/1 -> AntA Buffer/2(Q), AntB Buffer/2(Q)
  I/1 -> AntA Buffer/1(I), AntB Buffer/1(I)
  Wr Addr/1 -> AntA Buffer/4(Wr Addr), AntB Buffer/4(Wr Addr)
  FFT Vout/1 -> Logical1/2, Logical2/2
  AntA Buffer/2(" Q") -> Bus Creator2/2
  AntA Buffer/1(" I") -> Bus Creator1/2
  AntB Buffer/2(" Q") -> Bus Creator2/1
  AntB Buffer/1(" I") -> Bus Creator1/1
  LSB/1 -> Inverter/1, Logical1/1
  Logical1/1 -> AntB Buffer/3(We)
  Logical2/1 -> AntA Buffer/3(We)
  SymIndex/1 -> LSB/1
  Rd Addr/1 -> AntA Buffer/5(Rd Addr), AntB Buffer/5(Rd Addr)

== system ofdm_txrx_supermimo/OFDM Rx MIMO/FFT & Chan Est/Symbol Counter ==
  note: SR Latch enables counter after the first symbol. This keeps
      | the symbol index at zero after the first FFT finishes so that
      | the symbol index, like xn and xk_index, is zero-indexed.
  FFT Done : Inport  OutDataType=sfix(16)  OutScaling=2^0
  Payload : Inport  Port=2  OutDataType=sfix(16)  OutScaling=2^0
  Counter : xbsIndex_r4/Counter  cnt_type=Free Running  cnt_to=Inf  operation=Up  start_count=0  cnt_by_val=1  arith_type=Unsigned  n_bits=ceil(log2(max_OFDM_symbols+1))  bin_pt=0  load_pin=off  rst=on  en=on  explicit_period=off  period=1  dbl_ovrd=off  use_behavioral_HDL=off  use_rpm=on  implementation=Fabric  block_type=counter
      settings: xl_area=[6 12 0 12 0 0 0]  block_version=VER_STRING_GOES_HERE
  Inverter : xbsIndex_r4/Inverter  en=off  latency=0  dbl_ovrd=off  block_type=inv
      settings: xl_area=[0 0 0 0 0 0 0]  block_version=VER_STRING_GOES_HERE
  Logical1 : xbsIndex_r4/Logical  logical_function=AND  inputs=2  en=off  latency=0  precision=Full  arith_type=Unsigned  n_bits=8  bin_pt=2  align_bp=on  dbl_ovrd=off  block_type=logical
      settings: xl_area=[0, 0, 0, 0, 0, 0, 0]  block_version=VER_STRING_GOES_HERE
  Logical2 : xbsIndex_r4/Logical  logical_function=OR  inputs=2  en=off  latency=0  precision=Full  arith_type=Unsigned  n_bits=8  bin_pt=2  align_bp=on  dbl_ovrd=off  block_type=logical
      settings: xl_area=[4 0 0 4 0 0 0]  block_version=VER_STRING_GOES_HERE
  S-R Latch : Subsystem
  Up Sample : xbsIndex_r4/Up Sample  sample_ratio=4  copy_samples=on  dbl_ovrd=off  block_type=usamp
      settings: infoedit="Up samples input data.  Inserted values  can be zeros or copies of the most recent input sample.<P><P>Hardware notes: No hardware is needed if inserted values are copies of the input sample; otherwise, a mux and single bit flip-flop are used."  block_version=10.1.2
  Up Sample1 : xbsIndex_r4/Up Sample  sample_ratio=2  copy_samples=on  dbl_ovrd=off  block_type=usamp
      settings: infoedit="Up samples input data.  Inserted values  can be zeros or copies of the most recent input sample.<P><P>Hardware notes: No hardware is needed if inserted values are copies of the input sample; otherwise, a mux and single bit flip-flop are used."  block_version=10.1.2
  Sym Index : Outport  OutDataType=sfix(16)  OutScaling=2^0
  wires:
  Payload/1 -> Up Sample/1
  [rx_global_reset] -> Up Sample1/1
  Inverter/1 -> Logical2/1
  Logical2/1 -> S-R Latch/2(R), Counter/1
  S-R Latch/1(D) -> Logical1/2
  Logical1/1 -> Counter/2
  Counter/1 -> Sym Index/1
  FFT Done/1 -> S-R Latch/1(S), Logical1/1
  Up Sample/1 -> Inverter/1
  Up Sample1/1 -> Logical2/2

== system ofdm_txrx_supermimo/OFDM Rx MIMO/Long Corr HP/C1 ==
  x : Inport  OutDataType=sfix(16)  OutScaling=2^0
  AddSub : xbsIndex_r4/AddSub  Orientation=down  mode=Addition  use_carryin=off  use_carryout=off  en=off  latency=0  precision=Full  arith_type=Unsigned  n_bits=8  bin_pt=2  quantization=Truncate  overflow=Wrap  dbl_ovrd=off  use_behavioral_HDL=on  pipelined=off  use_rpm=off  hw_selection=Fabric  block_type=addsub
      settings: xl_area=[3 0 0 4 0 0 0]  block_version=VER_STRING_GOES_HERE
  AddSub{1..7,9..14} (13 alike) : xbsIndex_r4/AddSub  Orientation=down  mode=Addition  use_carryin=off  use_carryout=off  en=off  latency=1  precision=Full  arith_type=Unsigned  n_bits=8  bin_pt=2  quantization=Truncate  overflow=Wrap  dbl_ovrd=off  use_behavioral_HDL=on  pipelined=off  use_rpm=off  hw_selection=Fabric  block_type=addsub
      AddSub1 settings: xl_area=[3 5 0 4 0 0 0]  block_version=VER_STRING_GOES_HERE
      AddSub10 settings: xl_area=[3 5 0 4 0 0 0]  block_version=VER_STRING_GOES_HERE
      AddSub11 settings: xl_area=[3 5 0 4 0 0 0]  block_version=VER_STRING_GOES_HERE
      AddSub12 settings: xl_area=[3 5 0 4 0 0 0]  block_version=VER_STRING_GOES_HERE
      AddSub13 settings: xl_area=[3 6 0 5 0 0 0]  block_version=VER_STRING_GOES_HERE
      AddSub14 settings: xl_area=[3 6 0 5 0 0 0]  block_version=VER_STRING_GOES_HERE
      AddSub2 settings: xl_area=[3 6 0 5 0 0 0]  block_version=VER_STRING_GOES_HERE
      AddSub3 settings: xl_area=[3 5 0 4 0 0 0]  block_version=VER_STRING_GOES_HERE
      AddSub4 settings: xl_area=[4 7 0 6 0 0 0]  block_version=VER_STRING_GOES_HERE
      AddSub5 settings: xl_area=[3 5 0 4 0 0 0]  block_version=VER_STRING_GOES_HERE
      AddSub6 settings: xl_area=[3 6 0 5 0 0 0]  block_version=VER_STRING_GOES_HERE
      AddSub7 settings: xl_area=[3 5 0 4 0 0 0]  block_version=VER_STRING_GOES_HERE
      AddSub9 settings: xl_area=[4 7 0 6 0 0 0]  block_version=VER_STRING_GOES_HERE
  AddSub8 : xbsIndex_r4/AddSub  mode=Addition  use_carryin=off  use_carryout=off  en=off  latency=1  precision=Full  arith_type=Unsigned  n_bits=8  bin_pt=2  quantization=Truncate  overflow=Wrap  dbl_ovrd=off  use_behavioral_HDL=on  pipelined=off  use_rpm=off  hw_selection=Fabric  block_type=addsub
      settings: xl_area=[4 8 0 7 0 0 0]  block_version=VER_STRING_GOES_HERE
  C1 : Subsystem  mask{coef2=h(1:N)}
      mask prompts: coef2 "Coefficients"
  C10 : Subsystem  mask{coef2=h(9*N+1:10*N)}
      mask prompts: coef2 "Coefficients"
  C11 : Subsystem  mask{coef2=h(10*N+1:11*N)}
      mask prompts: coef2 "Coefficients"
  C12 : Subsystem  mask{coef2=h(11*N+1:12*N)}
      mask prompts: coef2 "Coefficients"
  C13 : Subsystem  mask{coef2=h(12*N+1:13*N)}
      mask prompts: coef2 "Coefficients"
  C14 : Subsystem  mask{coef2=h(13*N+1:14*N)}
      mask prompts: coef2 "Coefficients"
  C15 : Subsystem  mask{coef2=h(14*N+1:15*N)}
      mask prompts: coef2 "Coefficients"
  C16 : Subsystem  mask{coef2=h(15*N+1:16*N)}
      mask prompts: coef2 "Coefficients"
  C2 : Subsystem  mask{coef2=h(N+1:2*N)}
      mask prompts: coef2 "Coefficients"
  C3 : Subsystem  mask{coef2=h(2*N+1:3*N)}
      mask prompts: coef2 "Coefficients"
  C4 : Subsystem  mask{coef2=h(3*N+1:4*N)}
      mask prompts: coef2 "Coefficients"
  C5 : Subsystem  mask{coef2=h(4*N+1:5*N)}
      mask prompts: coef2 "Coefficients"
  C6 : Subsystem  mask{coef2=h(5*N+1:6*N)}
      mask prompts: coef2 "Coefficients"
  C7 : Subsystem  mask{coef2=h(6*N+1:7*N)}
      mask prompts: coef2 "Coefficients"
  C8 : Subsystem  mask{coef2=h(7*N+1:8*N)}
      mask prompts: coef2 "Coefficients"
  C9 : Subsystem  mask{coef2=h(8*N+1:9*N)}
      mask prompts: coef2 "Coefficients"
  Delay{1,3..4,6,8,12..13,15} (8 alike) : xbsIndex_r4/Delay  Orientation=down  en=off  latency=N-1  dbl_ovrd=off  reg_retiming=off  block_type=delay
      settings: infoedit=Hardware notes: A delay line is a chain, each link of which is an SRL16 followed by a flip-flop. If register retiming is enabled, the delay line is a chain of flip-flops.  xl_area=[2 4 0 4 0 0 0]  block_version=VER_STRING_GOES_HERE
  Delay10 : xbsIndex_r4/Delay  Orientation=down  en=off  latency=((N-1)*12)-1  dbl_ovrd=off  reg_retiming=off  block_type=delay
      settings: infoedit=Hardware notes: A delay line is a chain, each link of which is an SRL16 followed by a flip-flop. If register retiming is enabled, the delay line is a chain of flip-flops.  xl_area=[9 15 0 15 0 0 0]  block_version=VER_STRING_GOES_HERE
  Delay11 : xbsIndex_r4/Delay  Orientation=down  en=off  latency=((N-1)*14)-1  dbl_ovrd=off  reg_retiming=off  block_type=delay
      settings: infoedit=Hardware notes: A delay line is a chain, each link of which is an SRL16 followed by a flip-flop. If register retiming is enabled, the delay line is a chain of flip-flops.  xl_area=[9 15 0 15 0 0 0]  block_version=VER_STRING_GOES_HERE
  Delay14 : xbsIndex_r4/Delay  Orientation=down  en=off  latency=((N-1)*2)-1  dbl_ovrd=off  reg_retiming=off  block_type=delay
      settings: infoedit=Hardware notes: A delay line is a chain, each link of which is an SRL16 followed by a flip-flop. If register retiming is enabled, the delay line is a chain of flip-flops.  xl_area=[3 5 0 5 0 0 0]  block_version=VER_STRING_GOES_HERE
  Delay2 : xbsIndex_r4/Delay  Orientation=down  en=off  latency=((N-1)*4)-1  dbl_ovrd=off  reg_retiming=off  block_type=delay
      settings: infoedit=Hardware notes: A delay line is a chain, each link of which is an SRL16 followed by a flip-flop. If register retiming is enabled, the delay line is a chain of flip-flops.  xl_area=[3 5 0 5 0 0 0]  block_version=VER_STRING_GOES_HERE
  Delay5 : xbsIndex_r4/Delay  Orientation=down  en=off  latency=((N-1)*6)-1  dbl_ovrd=off  reg_retiming=off  block_type=delay
      settings: infoedit=Hardware notes: A delay line is a chain, each link of which is an SRL16 followed by a flip-flop. If register retiming is enabled, the delay line is a chain of flip-flops.  xl_area=[6 10 0 10 0 0 0]  block_version=VER_STRING_GOES_HERE
  Delay7 : xbsIndex_r4/Delay  Orientation=down  en=off  latency=((N-1)*8)-1  dbl_ovrd=off  reg_retiming=off  block_type=delay
      settings: infoedit=Hardware notes: A delay line is a chain, each link of which is an SRL16 followed by a flip-flop. If register retiming is enabled, the delay line is a chain of flip-flops.  xl_area=[6 10 0 10 0 0 0]  block_version=VER_STRING_GOES_HERE
  Delay9 : xbsIndex_r4/Delay  Orientation=down  en=off  latency=((N-1)*10)-1  dbl_ovrd=off  reg_retiming=off  block_type=delay
      settings: infoedit=Hardware notes: A delay line is a chain, each link of which is an SRL16 followed by a flip-flop. If register retiming is enabled, the delay line is a chain of flip-flops.  xl_area=[9 15 0 15 0 0 0]  block_version=VER_STRING_GOES_HERE
  FSM : Subsystem
  Gateway Out6 : xbsIndex_r4/Gateway Out  hdl_port=off  timing_constraint=None  locs_specified=off  LOCs={}  block_type=gatewayout
      settings: infoedit="Gateway out block.  Converts Xilinx fixed point inputs into ouputs of type Simulink integer, double, or fixed point.<P><P>Hardware notes:  In hardware these blocks become top level output ports or are discarded, depending on how they are configured."  xl_area=[0 0 0 0 0 0 0]  block_version=VER_STRING_GOES_HERE
  Gateway Out7 : xbsIndex_r4/Gateway Out  hdl_port=off  timing_constraint=None  locs_specified=off  LOCs={}  block_type=gatewayout
      settings: infoedit="Gateway out block.  Converts Xilinx fixed point inputs into ouputs of type Simulink integer, double, or fixed point.<P><P>Hardware notes:  In hardware these blocks become top level output ports or are discarded, depending on how they are configured."  xl_area=[0 0 0 0 0 0 0]  block_version=VER_STRING_GOES_HERE
  Gateway Out8 : xbsIndex_r4/Gateway Out  hdl_port=off  timing_constraint=None  locs_specified=off  LOCs={}  block_type=gatewayout
      settings: infoedit="Gateway out block.  Converts Xilinx fixed point inputs into ouputs of type Simulink integer, double, or fixed point.<P><P>Hardware notes:  In hardware these blocks become top level output ports or are discarded, depending on how they are configured."  xl_area=[0 0 0 0 0 0 0]  block_version=VER_STRING_GOES_HERE
  Long Corr Scope2 : Scope  NumInputPorts=3
      settings: YMin=-0.35~-0.175~0  YMax=0.35~0.225~1300  SaveName=ScopeData8
  y : Outport  OutDataType=sfix(16)  OutScaling=2^0
  wires:
  AddSub9/1 -> Gateway Out8/1, AddSub8/2
  AddSub6/1 -> AddSub9/1, Gateway Out7/1
  C13/1(yn) -> AddSub10/1
  AddSub8/1 -> y/1
  Delay9/1 -> AddSub6/2
  Delay7/1 -> AddSub6/1
  C12/1(yn) -> Delay8/1
  AddSub7/1 -> Delay9/1
  Delay8/1 -> AddSub7/2
  C11/1(yn) -> AddSub7/1
  AddSub4/1 -> AddSub8/1
  AddSub2/1 -> AddSub4/2
  AddSub13/1 -> AddSub4/1
  Delay5/1 -> AddSub2/2
  Delay2/1 -> AddSub2/1
  AddSub/1 -> AddSub13/1
  Delay14/1 -> AddSub13/2
  C8/2(xnz) -> [xnz]
  C10/1(yn) -> Delay6/1
  AddSub5/1 -> Delay7/1
  Delay6/1 -> AddSub5/2
  C9/1(yn) -> AddSub5/1
  C8/1(yn) -> Delay4/1
  C7/1(yn) -> AddSub3/1
  AddSub3/1 -> Delay5/1
  Delay4/1 -> AddSub3/2
  C7/2(xnz) -> C8/1(xn)
  C6/1(yn) -> Delay1/1
  AddSub1/1 -> Delay2/1
  Delay1/1 -> AddSub1/2
  C5/1(yn) -> AddSub1/1
  C1/1(yn) -> AddSub/1
  Delay3/1 -> AddSub/2
  AddSub12/1 -> Delay14/1
  Delay13/1 -> AddSub12/2
  C12/2(xnz) -> C13/1(xn)
  C11/2(xnz) -> C12/1(xn)
  C10/2(xnz) -> C11/1(xn)
  C9/2(xnz) -> C10/1(xn)
  [xnz] -> C9/1(xn)
  C6/2(xnz) -> C7/1(xn)
  C5/2(xnz) -> C6/1(xn)
  C4/1(yn) -> Delay13/1
  C4/2(xnz) -> C5/1(xn)
  C3/1(yn) -> AddSub12/1
  C3/2(xnz) -> C4/1(xn)
  C2/2(xnz) -> C3/1(xn)
  FSM/3(load) -> C12/4(LD), C13/4(LD), C14/4(LD), C16/4(LD), C15/4(LD), C11/4(LD), C10/4(LD), C9/4(LD), C1/4(LD), C8/4(LD), C7/4(LD), C6/4(LD), C5/4(LD), C4/4(LD), C3/4(LD), C2/4(LD)
  FSM/2(Coef Addr) -> C12/3(CAddr), C13/3(CAddr), C14/3(CAddr), C16/3(CAddr), C15/3(CAddr), C11/3(CAddr), C10/3(CAddr), C9/3(CAddr), C1/3(CAddr), C8/3(CAddr), C7/3(CAddr), C6/3(CAddr), C5/3(CAddr), C4/3(CAddr), C3/3(CAddr), C2/3(CAddr)
  FSM/1(Data Addr) -> C12/2(DAddr), C13/2(DAddr), C14/2(DAddr), C16/2(DAddr), C15/2(DAddr), C11/2(DAddr), C10/2(DAddr), C9/2(DAddr), C1/2(DAddr), C8/2(DAddr), C7/2(DAddr), C6/2(DAddr), C5/2(DAddr), C4/2(DAddr), C3/2(DAddr), C2/2(DAddr)
  C1/2(xnz) -> C2/1(xn)
  C2/1(yn) -> Delay3/1
  x/1 -> C1/1(xn)
  C13/2(xnz) -> C14/1(xn)
  C14/2(xnz) -> C15/1(xn)
  C15/2(xnz) -> C16/1(xn)
  C15/1(yn) -> AddSub11/1
  C14/1(yn) -> Delay12/1
  C16/1(yn) -> Delay15/1
  Delay12/1 -> AddSub10/2
  Delay15/1 -> AddSub11/2
  AddSub10/1 -> Delay10/1
  AddSub11/1 -> Delay11/1
  Delay10/1 -> AddSub14/1
  Delay11/1 -> AddSub14/2
  AddSub14/1 -> AddSub9/2, Gateway Out6/1
  Gateway Out6/1 -> Long Corr Scope2/1
  Gateway Out7/1 -> Long Corr Scope2/2
  Gateway Out8/1 -> Long Corr Scope2/3
